simulink model "rfi_blk_v6_casper"  (Simulink 7.1)
settings: Solver=ode45  StartTime=0.0  StopTime=10.0  RelTol=1e-3
undefined symbols (not defined in the file): a, b
libraries: xbsIndex_r4 x13084, xps_library x4
(part 2 of 51)

== system rfi_blk_v6_casper/rfi_blk/mad_comp/sync_control ==
  note: 1K window acc_reset signal
  pps : Inport
  Constant : xbsIndex_r4/Constant  arith_type=Unsigned  const=1021  n_bits=10  bin_pt=0  explicit_period=on  period=1  equ=P=C  opselect=C  inp2=PCIN>>17  opr=+  inp1=P  carry=CIN  dbl_ovrd=off  block_type=constant
  Convert1 : xbsIndex_r4/Convert  arith_type=Boolean  n_bits=16  bin_pt=14  quantization=Truncate  overflow=Wrap  latency=0  dbl_ovrd=off  pipeline=off  block_type=convert
      settings: block_version=10.1.3
  Convert3 : xbsIndex_r4/Convert  arith_type=Boolean  n_bits=16  bin_pt=14  quantization=Truncate  overflow=Wrap  latency=0  dbl_ovrd=off  pipeline=off  block_type=convert
      settings: block_version=10.1.3
  Counter2 : xbsIndex_r4/Counter  cnt_type=Free Running  cnt_to=2048  operation=Up  start_count=0  cnt_by_val=1  arith_type=Unsigned  n_bits=10  bin_pt=0  load_pin=off  rst=off  en=off  explicit_period=on  period=1  dbl_ovrd=off  use_behavioral_HDL=off  implementation=Fabric  use_rpm=off  block_type=counter
  Delay2 : xbsIndex_r4/Delay  en=off  latency=1  dbl_ovrd=off  reg_retiming=off  block_type=delay
  Logical : xbsIndex_r4/Logical  logical_function=OR  inputs=2  en=off  latency=0  precision=Full  arith_type=Unsigned  n_bits=16  bin_pt=0  align_bp=on  dbl_ovrd=off  block_type=logical
  Relational : xbsIndex_r4/Relational  mode=a=b  en=off  latency=1  dbl_ovrd=off  block_type=relational
      settings: block_version=10.1.3
  Out1 : Outport
  wires:
  Counter2/1 -> Relational/1
  Constant/1 -> Relational/2
  Relational/1 -> Convert3/1
  Convert3/1 -> Logical/2
  Logical/1 -> Out1/1
  pps/1 -> Delay2/1
  Convert1/1 -> Logical/1
  Delay2/1 -> Convert1/1

== system rfi_blk_v6_casper/rfi_blk/cr1/cr/clip_repl ==
  note: 0: Bypass
      | 1: Replace by constant value
      | 2: Replace by median value
      | 3: Replace with Digital Noise
  p_thres : Inport  OutDataType=sfix(16)  OutScaling=2^0
  n_thres : Inport  Port=2  OutDataType=sfix(16)  OutScaling=2^0
  input : Inport  Port=3  OutDataType=sfix(16)  OutScaling=2^0
  median : Inport  Port=4  OutDataType=sfix(16)  OutScaling=2^0
  sigma_mad : Inport  Port=5  OutDataType=sfix(16)  OutScaling=2^0
  repl_val : Inport  Port=6  OutDataType=sfix(16)  OutScaling=2^0
  bypass_mux : Inport  Port=7  OutDataType=sfix(16)  OutScaling=2^0
  Delay1 : xbsIndex_r4/Delay  en=off  latency=3  dbl_ovrd=off  reg_retiming=off  block_type=delay
  Delay2 : xbsIndex_r4/Delay  en=off  latency=2  dbl_ovrd=off  reg_retiming=off  block_type=delay
  Delay3 : xbsIndex_r4/Delay  en=off  latency=2  dbl_ovrd=off  reg_retiming=off  block_type=delay
  Logical : xbsIndex_r4/Logical  logical_function=OR  inputs=2  en=off  latency=1  precision=Full  arith_type="Signed  (2's comp)"  n_bits=8  bin_pt=0  align_bp=on  dbl_ovrd=off  block_type=logical
  Mux : xbsIndex_r4/Mux  inputs=2  en=off  latency=1  precision=User Defined  arith_type="Signed  (2's comp)"  n_bits=8  bin_pt=0  quantization=Truncate  overflow=Wrap  dbl_ovrd=off  block_type=mux
  Mux1 : xbsIndex_r4/Mux  inputs=4  en=off  latency=1  precision=User Defined  arith_type="Signed  (2's comp)"  n_bits=8  bin_pt=0  quantization=Truncate  overflow=Wrap  dbl_ovrd=off  block_type=mux
  Mux2 : xbsIndex_r4/Mux  inputs=2  en=off  latency=1  precision=Full  arith_type="Signed  (2's comp)"  n_bits=8  bin_pt=0  quantization=Truncate  overflow=Wrap  dbl_ovrd=off  block_type=mux
  Mux3 : xbsIndex_r4/Mux  inputs=2  en=off  latency=1  precision=Full  arith_type="Signed  (2's comp)"  n_bits=8  bin_pt=0  quantization=Truncate  overflow=Wrap  dbl_ovrd=off  block_type=mux
  Relational : xbsIndex_r4/Relational  mode=a>b  en=off  latency=1  dbl_ovrd=off  block_type=relational
      settings: block_version=10.1.3
  Relational1 : xbsIndex_r4/Relational  mode=a<b  en=off  latency=1  dbl_ovrd=off  block_type=relational
      settings: block_version=10.1.3
  dns : Subsystem
  filter_o : Outport
  detect_o : Outport  Port=2
  input_data : Outport  Port=3
  wires:
  p_thres/1 -> Relational/2
  n_thres/1 -> Relational1/2
  Relational/1 -> Logical/1
  Relational1/1 -> Logical/2
  Logical/1 -> Mux/1, Mux2/1, Mux3/1, Delay2/1
  Delay1/1 -> Mux/2, Mux3/2, Mux1/2, Mux2/2, Delay3/1
  input/1 -> Relational/1, Relational1/1, Delay1/1
  Mux/1 -> Mux1/3
  Mux1/1 -> filter_o/1
  Mux3/1 -> Mux1/4
  Mux2/1 -> Mux1/5
  median/1 -> Mux3/3
  dns/1(dns_o) -> Mux2/3
  sigma_mad/1 -> dns/1(sigma_mad)
  Delay2/1 -> detect_o/1
  Delay3/1 -> input_data/1
  repl_val/1 -> Mux/3
  bypass_mux/1 -> Mux1/1

== system rfi_blk_v6_casper/rfi_blk/cr1/cr/thres_calc ==
  mad : Inport
  median : Inport  Port=2
  thres_fact : Inport  Port=3
  AddSub : xbsIndex_r4/AddSub  mode=Addition  use_carryin=off  use_carryout=off  en=off  latency=0  precision=Full  arith_type="Signed  (2's comp)"  n_bits=8  bin_pt=0  quantization=Truncate  overflow=Wrap  dbl_ovrd=off  use_behavioral_HDL=off  hw_selection=Fabric  pipelined=off  use_rpm=on  block_type=addsub
      settings: block_version=10.1.3
  AddSub1 : xbsIndex_r4/AddSub  mode=Subtraction  use_carryin=off  use_carryout=off  en=off  latency=0  precision=Full  arith_type="Signed  (2's comp)"  n_bits=8  bin_pt=0  quantization=Truncate  overflow=Wrap  dbl_ovrd=off  use_behavioral_HDL=off  hw_selection=Fabric  pipelined=off  use_rpm=on  block_type=addsub
      settings: block_version=10.1.3
  Constant : xbsIndex_r4/Constant  arith_type=Unsigned  const=1.4826  n_bits=8  bin_pt=7  explicit_period=on  period=1  equ=P=C  opselect=C  inp2=PCIN>>17  opr=+  inp1=P  carry=CIN  dbl_ovrd=off  block_type=constant
  Mult : xbsIndex_r4/Mult  precision=Full  arith_type="Signed  (2's comp)"  n_bits=16  bin_pt=14  quantization=Truncate  overflow=Wrap  en=off  latency=3  dbl_ovrd=off  use_behavioral_HDL=off  opt=Speed  use_embedded=on  optimum_pipeline=off  pipeline=on  use_rpm=on  placement_style=Triangular  block_type=mult
      settings: infoedit=Hardware notes: To use the internal pipeline stage of the dedicated multiplier you must select 'Pipeline for maximum performance'.  block_version=10.1.3
  Mult1 : xbsIndex_r4/Mult  precision=Full  arith_type=Unsigned  n_bits=8  bin_pt=0  quantization=Truncate  overflow=Wrap  en=off  latency=3  dbl_ovrd=off  use_behavioral_HDL=off  opt=Speed  use_embedded=on  optimum_pipeline=off  pipeline=on  use_rpm=on  placement_style=Triangular  block_type=mult
      settings: block_version=10.1.3
  Reinterpret : xbsIndex_r4/Reinterpret  force_arith_type=on  arith_type=Unsigned  force_bin_pt=off  bin_pt=0  block_type=reinterpret
      settings: infoedit="Changes signal type without altering the binary representation.   You can changed the signal between signed and unsigned, and relocate the binary point.<br><br>Hardware notes: In hardware this block costs nothing.<br><br>Example:  Suppose the input is 6 bits wide, signed, with 2 fractional bits, and the output is forced to unsigned with 0 fractional bits.  Then an input of -2.0 (1110.00 in binary 2's complement) becomes an output of 56 (111000 in binary)."  block_version=10.1.3
  Reinterpret1 : xbsIndex_r4/Reinterpret  force_arith_type=on  arith_type=Unsigned  force_bin_pt=off  bin_pt=0  block_type=reinterpret
      settings: infoedit="Changes signal type without altering the binary representation.   You can changed the signal between signed and unsigned, and relocate the binary point.<br><br>Hardware notes: In hardware this block costs nothing.<br><br>Example:  Suppose the input is 6 bits wide, signed, with 2 fractional bits, and the output is forced to unsigned with 0 fractional bits.  Then an input of -2.0 (1110.00 in binary 2's complement) becomes an output of 56 (111000 in binary)."  block_version=10.1.3
  Reinterpret2 : xbsIndex_r4/Reinterpret  force_arith_type=on  arith_type="Signed  (2's comp)"  force_bin_pt=off  bin_pt=0  block_type=reinterpret
      settings: infoedit="Changes signal type without altering the binary representation.   You can changed the signal between signed and unsigned, and relocate the binary point.<br><br>Hardware notes: In hardware this block costs nothing.<br><br>Example:  Suppose the input is 6 bits wide, signed, with 2 fractional bits, and the output is forced to unsigned with 0 fractional bits.  Then an input of -2.0 (1110.00 in binary 2's complement) becomes an output of 56 (111000 in binary)."  block_version=10.1.3
  Scope1 : Scope  NumInputPorts=5
      settings: YMin=-5~-5~-5~-5~-5  YMax=5~5~5~5~5  SaveName=ScopeData26
  Scope3 : Scope  NumInputPorts=5
      settings: YMin=-5~-5~-5~-5~-5  YMax=5~5~5~5~5  SaveName=ScopeData20
  p_th : Outport
  n_th : Outport  Port=2
  sigma_mad_o : Outport  Port=3
  wires:
  Constant/1 -> Mult/2
  Mult/1 -> Reinterpret/1
  Reinterpret/1 -> Mult1/1, sigma_mad_o/1
  Mult1/1 -> Reinterpret1/1
  mad/1 -> Mult/1, Scope3/2
  median/1 -> Reinterpret2/1, Scope3/1
  Reinterpret1/1 -> AddSub/2, AddSub1/2
  AddSub/1 -> p_th/1, Scope1/1
  AddSub1/1 -> n_th/1, Scope1/2
  Reinterpret2/1 -> AddSub/1, AddSub1/1
  thres_fact/1 -> Mult1/2

== system rfi_blk_v6_casper/rfi_blk/cr2/cr/clip_repl ==
  note: 0: Bypass
      | 1: Replace by constant value
      | 2: Replace by median value
      | 3: Replace with Digital Noise
  p_thres : Inport  OutDataType=sfix(16)  OutScaling=2^0
  n_thres : Inport  Port=2  OutDataType=sfix(16)  OutScaling=2^0
  input : Inport  Port=3  OutDataType=sfix(16)  OutScaling=2^0
  median : Inport  Port=4  OutDataType=sfix(16)  OutScaling=2^0
  sigma_mad : Inport  Port=5  OutDataType=sfix(16)  OutScaling=2^0
  repl_val : Inport  Port=6  OutDataType=sfix(16)  OutScaling=2^0
  bypass_mux : Inport  Port=7  OutDataType=sfix(16)  OutScaling=2^0
  Delay1 : xbsIndex_r4/Delay  en=off  latency=3  dbl_ovrd=off  reg_retiming=off  block_type=delay
  Delay2 : xbsIndex_r4/Delay  en=off  latency=2  dbl_ovrd=off  reg_retiming=off  block_type=delay
  Delay3 : xbsIndex_r4/Delay  en=off  latency=2  dbl_ovrd=off  reg_retiming=off  block_type=delay
  Logical : xbsIndex_r4/Logical  logical_function=OR  inputs=2  en=off  latency=1  precision=Full  arith_type="Signed  (2's comp)"  n_bits=8  bin_pt=0  align_bp=on  dbl_ovrd=off  block_type=logical
  Mux : xbsIndex_r4/Mux  inputs=2  en=off  latency=1  precision=User Defined  arith_type="Signed  (2's comp)"  n_bits=8  bin_pt=0  quantization=Truncate  overflow=Wrap  dbl_ovrd=off  block_type=mux
  Mux1 : xbsIndex_r4/Mux  inputs=4  en=off  latency=1  precision=User Defined  arith_type="Signed  (2's comp)"  n_bits=8  bin_pt=0  quantization=Truncate  overflow=Wrap  dbl_ovrd=off  block_type=mux
  Mux2 : xbsIndex_r4/Mux  inputs=2  en=off  latency=1  precision=Full  arith_type="Signed  (2's comp)"  n_bits=8  bin_pt=0  quantization=Truncate  overflow=Wrap  dbl_ovrd=off  block_type=mux
  Mux3 : xbsIndex_r4/Mux  inputs=2  en=off  latency=1  precision=Full  arith_type="Signed  (2's comp)"  n_bits=8  bin_pt=0  quantization=Truncate  overflow=Wrap  dbl_ovrd=off  block_type=mux
  Relational : xbsIndex_r4/Relational  mode=a>b  en=off  latency=1  dbl_ovrd=off  block_type=relational
      settings: block_version=10.1.3
  Relational1 : xbsIndex_r4/Relational  mode=a<b  en=off  latency=1  dbl_ovrd=off  block_type=relational
      settings: block_version=10.1.3
  dns : Subsystem
  filter_o : Outport
  detect_o : Outport  Port=2
  input_data : Outport  Port=3
  wires:
  p_thres/1 -> Relational/2
  n_thres/1 -> Relational1/2
  Relational/1 -> Logical/1
  Relational1/1 -> Logical/2
  Logical/1 -> Mux/1, Mux2/1, Mux3/1, Delay2/1
  Delay1/1 -> Mux/2, Mux3/2, Mux1/2, Mux2/2, Delay3/1
  input/1 -> Relational/1, Relational1/1, Delay1/1
  Mux/1 -> Mux1/3
  Mux1/1 -> filter_o/1
  Mux3/1 -> Mux1/4
  Mux2/1 -> Mux1/5
  median/1 -> Mux3/3
  dns/1(dns_o) -> Mux2/3
  sigma_mad/1 -> dns/1(sigma_mad)
  Delay2/1 -> detect_o/1
  Delay3/1 -> input_data/1
  repl_val/1 -> Mux/3
  bypass_mux/1 -> Mux1/1

== system rfi_blk_v6_casper/rfi_blk/cr2/cr/thres_calc ==
  mad : Inport
  median : Inport  Port=2
  thres_fact : Inport  Port=3
  AddSub : xbsIndex_r4/AddSub  mode=Addition  use_carryin=off  use_carryout=off  en=off  latency=0  precision=Full  arith_type="Signed  (2's comp)"  n_bits=8  bin_pt=0  quantization=Truncate  overflow=Wrap  dbl_ovrd=off  use_behavioral_HDL=off  hw_selection=Fabric  pipelined=off  use_rpm=on  block_type=addsub
      settings: block_version=10.1.3
  AddSub1 : xbsIndex_r4/AddSub  mode=Subtraction  use_carryin=off  use_carryout=off  en=off  latency=0  precision=Full  arith_type="Signed  (2's comp)"  n_bits=8  bin_pt=0  quantization=Truncate  overflow=Wrap  dbl_ovrd=off  use_behavioral_HDL=off  hw_selection=Fabric  pipelined=off  use_rpm=on  block_type=addsub
      settings: block_version=10.1.3
  Constant : xbsIndex_r4/Constant  arith_type=Unsigned  const=1.4826  n_bits=8  bin_pt=7  explicit_period=on  period=1  equ=P=C  opselect=C  inp2=PCIN>>17  opr=+  inp1=P  carry=CIN  dbl_ovrd=off  block_type=constant
  Mult : xbsIndex_r4/Mult  precision=Full  arith_type="Signed  (2's comp)"  n_bits=16  bin_pt=14  quantization=Truncate  overflow=Wrap  en=off  latency=3  dbl_ovrd=off  use_behavioral_HDL=off  opt=Speed  use_embedded=on  optimum_pipeline=off  pipeline=on  use_rpm=on  placement_style=Triangular  block_type=mult
      settings: infoedit=Hardware notes: To use the internal pipeline stage of the dedicated multiplier you must select 'Pipeline for maximum performance'.  block_version=10.1.3
  Mult1 : xbsIndex_r4/Mult  precision=Full  arith_type=Unsigned  n_bits=8  bin_pt=0  quantization=Truncate  overflow=Wrap  en=off  latency=3  dbl_ovrd=off  use_behavioral_HDL=off  opt=Speed  use_embedded=on  optimum_pipeline=off  pipeline=on  use_rpm=on  placement_style=Triangular  block_type=mult
      settings: block_version=10.1.3
  Reinterpret : xbsIndex_r4/Reinterpret  force_arith_type=on  arith_type=Unsigned  force_bin_pt=off  bin_pt=0  block_type=reinterpret
      settings: infoedit="Changes signal type without altering the binary representation.   You can changed the signal between signed and unsigned, and relocate the binary point.<br><br>Hardware notes: In hardware this block costs nothing.<br><br>Example:  Suppose the input is 6 bits wide, signed, with 2 fractional bits, and the output is forced to unsigned with 0 fractional bits.  Then an input of -2.0 (1110.00 in binary 2's complement) becomes an output of 56 (111000 in binary)."  block_version=10.1.3
  Reinterpret1 : xbsIndex_r4/Reinterpret  force_arith_type=on  arith_type=Unsigned  force_bin_pt=off  bin_pt=0  block_type=reinterpret
      settings: infoedit="Changes signal type without altering the binary representation.   You can changed the signal between signed and unsigned, and relocate the binary point.<br><br>Hardware notes: In hardware this block costs nothing.<br><br>Example:  Suppose the input is 6 bits wide, signed, with 2 fractional bits, and the output is forced to unsigned with 0 fractional bits.  Then an input of -2.0 (1110.00 in binary 2's complement) becomes an output of 56 (111000 in binary)."  block_version=10.1.3
  Reinterpret2 : xbsIndex_r4/Reinterpret  force_arith_type=on  arith_type="Signed  (2's comp)"  force_bin_pt=off  bin_pt=0  block_type=reinterpret
      settings: infoedit="Changes signal type without altering the binary representation.   You can changed the signal between signed and unsigned, and relocate the binary point.<br><br>Hardware notes: In hardware this block costs nothing.<br><br>Example:  Suppose the input is 6 bits wide, signed, with 2 fractional bits, and the output is forced to unsigned with 0 fractional bits.  Then an input of -2.0 (1110.00 in binary 2's complement) becomes an output of 56 (111000 in binary)."  block_version=10.1.3
  Scope1 : Scope  NumInputPorts=5
      settings: YMin=-5~-5~-5~-5~-5  YMax=5~5~5~5~5  SaveName=ScopeData26
  Scope3 : Scope  NumInputPorts=5
      settings: YMin=-5~-5~-5~-5~-5  YMax=5~5~5~5~5  SaveName=ScopeData20
  p_th : Outport
  n_th : Outport  Port=2
  sigma_mad_o : Outport  Port=3
  wires:
  Constant/1 -> Mult/2
  Mult/1 -> Reinterpret/1
  Reinterpret/1 -> Mult1/1, sigma_mad_o/1
  Mult1/1 -> Reinterpret1/1
  mad/1 -> Mult/1, Scope3/2
  median/1 -> Reinterpret2/1, Scope3/1
  Reinterpret1/1 -> AddSub/2, AddSub1/2
  AddSub/1 -> p_th/1, Scope1/1
  AddSub1/1 -> n_th/1, Scope1/2
  Reinterpret2/1 -> AddSub/1, AddSub1/1
  thres_fact/1 -> Mult1/2

== system rfi_blk_v6_casper/rfi_blk/cr4/cr/clip_repl ==
  note: 0: Bypass
      | 1: Replace by constant value
      | 2: Replace by median value
      | 3: Replace with Digital Noise
  p_thres : Inport  OutDataType=sfix(16)  OutScaling=2^0
  n_thres : Inport  Port=2  OutDataType=sfix(16)  OutScaling=2^0
  input : Inport  Port=3  OutDataType=sfix(16)  OutScaling=2^0
  median : Inport  Port=4  OutDataType=sfix(16)  OutScaling=2^0
  sigma_mad : Inport  Port=5  OutDataType=sfix(16)  OutScaling=2^0
  repl_val : Inport  Port=6  OutDataType=sfix(16)  OutScaling=2^0
  bypass_mux : Inport  Port=7  OutDataType=sfix(16)  OutScaling=2^0
  Delay1 : xbsIndex_r4/Delay  en=off  latency=3  dbl_ovrd=off  reg_retiming=off  block_type=delay
  Delay2 : xbsIndex_r4/Delay  en=off  latency=2  dbl_ovrd=off  reg_retiming=off  block_type=delay
  Delay3 : xbsIndex_r4/Delay  en=off  latency=2  dbl_ovrd=off  reg_retiming=off  block_type=delay
  Logical : xbsIndex_r4/Logical  logical_function=OR  inputs=2  en=off  latency=1  precision=Full  arith_type="Signed  (2's comp)"  n_bits=8  bin_pt=0  align_bp=on  dbl_ovrd=off  block_type=logical
  Mux : xbsIndex_r4/Mux  inputs=2  en=off  latency=1  precision=User Defined  arith_type="Signed  (2's comp)"  n_bits=8  bin_pt=0  quantization=Truncate  overflow=Wrap  dbl_ovrd=off  block_type=mux
  Mux1 : xbsIndex_r4/Mux  inputs=4  en=off  latency=1  precision=User Defined  arith_type="Signed  (2's comp)"  n_bits=8  bin_pt=0  quantization=Truncate  overflow=Wrap  dbl_ovrd=off  block_type=mux
  Mux2 : xbsIndex_r4/Mux  inputs=2  en=off  latency=1  precision=Full  arith_type="Signed  (2's comp)"  n_bits=8  bin_pt=0  quantization=Truncate  overflow=Wrap  dbl_ovrd=off  block_type=mux
  Mux3 : xbsIndex_r4/Mux  inputs=2  en=off  latency=1  precision=Full  arith_type="Signed  (2's comp)"  n_bits=8  bin_pt=0  quantization=Truncate  overflow=Wrap  dbl_ovrd=off  block_type=mux
  Relational : xbsIndex_r4/Relational  mode=a>b  en=off  latency=1  dbl_ovrd=off  block_type=relational
      settings: block_version=10.1.3
  Relational1 : xbsIndex_r4/Relational  mode=a<b  en=off  latency=1  dbl_ovrd=off  block_type=relational
      settings: block_version=10.1.3
  dns : Subsystem
  filter_o : Outport
  detect_o : Outport  Port=2
  input_data : Outport  Port=3
  wires:
  p_thres/1 -> Relational/2
  n_thres/1 -> Relational1/2
  Relational/1 -> Logical/1
  Relational1/1 -> Logical/2
  Logical/1 -> Mux/1, Mux2/1, Mux3/1, Delay2/1
  Delay1/1 -> Mux/2, Mux3/2, Mux1/2, Mux2/2, Delay3/1
  input/1 -> Relational/1, Relational1/1, Delay1/1
  Mux/1 -> Mux1/3
  Mux1/1 -> filter_o/1
  Mux3/1 -> Mux1/4
  Mux2/1 -> Mux1/5
  median/1 -> Mux3/3
  dns/1(dns_o) -> Mux2/3
  sigma_mad/1 -> dns/1(sigma_mad)
  Delay2/1 -> detect_o/1
  Delay3/1 -> input_data/1
  repl_val/1 -> Mux/3
  bypass_mux/1 -> Mux1/1

== system rfi_blk_v6_casper/rfi_blk/cr4/cr/thres_calc ==
  mad : Inport
  median : Inport  Port=2
  thres_fact : Inport  Port=3
  AddSub : xbsIndex_r4/AddSub  mode=Addition  use_carryin=off  use_carryout=off  en=off  latency=0  precision=Full  arith_type="Signed  (2's comp)"  n_bits=8  bin_pt=0  quantization=Truncate  overflow=Wrap  dbl_ovrd=off  use_behavioral_HDL=off  hw_selection=Fabric  pipelined=off  use_rpm=on  block_type=addsub
      settings: block_version=10.1.3
  AddSub1 : xbsIndex_r4/AddSub  mode=Subtraction  use_carryin=off  use_carryout=off  en=off  latency=0  precision=Full  arith_type="Signed  (2's comp)"  n_bits=8  bin_pt=0  quantization=Truncate  overflow=Wrap  dbl_ovrd=off  use_behavioral_HDL=off  hw_selection=Fabric  pipelined=off  use_rpm=on  block_type=addsub
      settings: block_version=10.1.3
  Constant : xbsIndex_r4/Constant  arith_type=Unsigned  const=1.4826  n_bits=8  bin_pt=7  explicit_period=on  period=1  equ=P=C  opselect=C  inp2=PCIN>>17  opr=+  inp1=P  carry=CIN  dbl_ovrd=off  block_type=constant
  Mult : xbsIndex_r4/Mult  precision=Full  arith_type="Signed  (2's comp)"  n_bits=16  bin_pt=14  quantization=Truncate  overflow=Wrap  en=off  latency=3  dbl_ovrd=off  use_behavioral_HDL=off  opt=Speed  use_embedded=on  optimum_pipeline=off  pipeline=on  use_rpm=on  placement_style=Triangular  block_type=mult
      settings: infoedit=Hardware notes: To use the internal pipeline stage of the dedicated multiplier you must select 'Pipeline for maximum performance'.  block_version=10.1.3
  Mult1 : xbsIndex_r4/Mult  precision=Full  arith_type=Unsigned  n_bits=8  bin_pt=0  quantization=Truncate  overflow=Wrap  en=off  latency=3  dbl_ovrd=off  use_behavioral_HDL=off  opt=Speed  use_embedded=on  optimum_pipeline=off  pipeline=on  use_rpm=on  placement_style=Triangular  block_type=mult
      settings: block_version=10.1.3
  Reinterpret : xbsIndex_r4/Reinterpret  force_arith_type=on  arith_type=Unsigned  force_bin_pt=off  bin_pt=0  block_type=reinterpret
      settings: infoedit="Changes signal type without altering the binary representation.   You can changed the signal between signed and unsigned, and relocate the binary point.<br><br>Hardware notes: In hardware this block costs nothing.<br><br>Example:  Suppose the input is 6 bits wide, signed, with 2 fractional bits, and the output is forced to unsigned with 0 fractional bits.  Then an input of -2.0 (1110.00 in binary 2's complement) becomes an output of 56 (111000 in binary)."  block_version=10.1.3
  Reinterpret1 : xbsIndex_r4/Reinterpret  force_arith_type=on  arith_type=Unsigned  force_bin_pt=off  bin_pt=0  block_type=reinterpret
      settings: infoedit="Changes signal type without altering the binary representation.   You can changed the signal between signed and unsigned, and relocate the binary point.<br><br>Hardware notes: In hardware this block costs nothing.<br><br>Example:  Suppose the input is 6 bits wide, signed, with 2 fractional bits, and the output is forced to unsigned with 0 fractional bits.  Then an input of -2.0 (1110.00 in binary 2's complement) becomes an output of 56 (111000 in binary)."  block_version=10.1.3
  Reinterpret2 : xbsIndex_r4/Reinterpret  force_arith_type=on  arith_type="Signed  (2's comp)"  force_bin_pt=off  bin_pt=0  block_type=reinterpret
      settings: infoedit="Changes signal type without altering the binary representation.   You can changed the signal between signed and unsigned, and relocate the binary point.<br><br>Hardware notes: In hardware this block costs nothing.<br><br>Example:  Suppose the input is 6 bits wide, signed, with 2 fractional bits, and the output is forced to unsigned with 0 fractional bits.  Then an input of -2.0 (1110.00 in binary 2's complement) becomes an output of 56 (111000 in binary)."  block_version=10.1.3
  Scope1 : Scope  NumInputPorts=5
      settings: YMin=-5~-5~-5~-5~-5  YMax=5~5~5~5~5  SaveName=ScopeData26
  Scope3 : Scope  NumInputPorts=5
      settings: YMin=-5~-5~-5~-5~-5  YMax=5~5~5~5~5  SaveName=ScopeData20
  p_th : Outport
  n_th : Outport  Port=2
  sigma_mad_o : Outport  Port=3
  wires:
  Constant/1 -> Mult/2
  Mult/1 -> Reinterpret/1
  Reinterpret/1 -> Mult1/1, sigma_mad_o/1
  Mult1/1 -> Reinterpret1/1
  mad/1 -> Mult/1, Scope3/2
  median/1 -> Reinterpret2/1, Scope3/1
  Reinterpret1/1 -> AddSub/2, AddSub1/2
  AddSub/1 -> p_th/1, Scope1/1
  AddSub1/1 -> n_th/1, Scope1/2
  Reinterpret2/1 -> AddSub/1, AddSub1/1
  thres_fact/1 -> Mult1/2

== system rfi_blk_v6_casper/rfi_blk/cr5/cr/clip_repl ==
  note: 0: Bypass
      | 1: Replace by constant value
      | 2: Replace by median value
      | 3: Replace with Digital Noise
  p_thres : Inport  OutDataType=sfix(16)  OutScaling=2^0
  n_thres : Inport  Port=2  OutDataType=sfix(16)  OutScaling=2^0
  input : Inport  Port=3  OutDataType=sfix(16)  OutScaling=2^0
  median : Inport  Port=4  OutDataType=sfix(16)  OutScaling=2^0
  sigma_mad : Inport  Port=5  OutDataType=sfix(16)  OutScaling=2^0
  repl_val : Inport  Port=6  OutDataType=sfix(16)  OutScaling=2^0
  bypass_mux : Inport  Port=7  OutDataType=sfix(16)  OutScaling=2^0
  Delay1 : xbsIndex_r4/Delay  en=off  latency=3  dbl_ovrd=off  reg_retiming=off  block_type=delay
  Delay2 : xbsIndex_r4/Delay  en=off  latency=2  dbl_ovrd=off  reg_retiming=off  block_type=delay
  Delay3 : xbsIndex_r4/Delay  en=off  latency=2  dbl_ovrd=off  reg_retiming=off  block_type=delay
  Logical : xbsIndex_r4/Logical  logical_function=OR  inputs=2  en=off  latency=1  precision=Full  arith_type="Signed  (2's comp)"  n_bits=8  bin_pt=0  align_bp=on  dbl_ovrd=off  block_type=logical
  Mux : xbsIndex_r4/Mux  inputs=2  en=off  latency=1  precision=User Defined  arith_type="Signed  (2's comp)"  n_bits=8  bin_pt=0  quantization=Truncate  overflow=Wrap  dbl_ovrd=off  block_type=mux
  Mux1 : xbsIndex_r4/Mux  inputs=4  en=off  latency=1  precision=User Defined  arith_type="Signed  (2's comp)"  n_bits=8  bin_pt=0  quantization=Truncate  overflow=Wrap  dbl_ovrd=off  block_type=mux
  Mux2 : xbsIndex_r4/Mux  inputs=2  en=off  latency=1  precision=Full  arith_type="Signed  (2's comp)"  n_bits=8  bin_pt=0  quantization=Truncate  overflow=Wrap  dbl_ovrd=off  block_type=mux
  Mux3 : xbsIndex_r4/Mux  inputs=2  en=off  latency=1  precision=Full  arith_type="Signed  (2's comp)"  n_bits=8  bin_pt=0  quantization=Truncate  overflow=Wrap  dbl_ovrd=off  block_type=mux
  Relational : xbsIndex_r4/Relational  mode=a>b  en=off  latency=1  dbl_ovrd=off  block_type=relational
      settings: block_version=10.1.3
  Relational1 : xbsIndex_r4/Relational  mode=a<b  en=off  latency=1  dbl_ovrd=off  block_type=relational
      settings: block_version=10.1.3
  dns : Subsystem
  filter_o : Outport
  detect_o : Outport  Port=2
  input_data : Outport  Port=3
  wires:
  p_thres/1 -> Relational/2
  n_thres/1 -> Relational1/2
  Relational/1 -> Logical/1
  Relational1/1 -> Logical/2
  Logical/1 -> Mux/1, Mux2/1, Mux3/1, Delay2/1
  Delay1/1 -> Mux/2, Mux3/2, Mux1/2, Mux2/2, Delay3/1
  input/1 -> Relational/1, Relational1/1, Delay1/1
  Mux/1 -> Mux1/3
  Mux1/1 -> filter_o/1
  Mux3/1 -> Mux1/4
  Mux2/1 -> Mux1/5
  median/1 -> Mux3/3
  dns/1(dns_o) -> Mux2/3
  sigma_mad/1 -> dns/1(sigma_mad)
  Delay2/1 -> detect_o/1
  Delay3/1 -> input_data/1
  repl_val/1 -> Mux/3
  bypass_mux/1 -> Mux1/1

== system rfi_blk_v6_casper/rfi_blk/cr5/cr/thres_calc ==
  mad : Inport
  median : Inport  Port=2
  thres_fact : Inport  Port=3
  AddSub : xbsIndex_r4/AddSub  mode=Addition  use_carryin=off  use_carryout=off  en=off  latency=0  precision=Full  arith_type="Signed  (2's comp)"  n_bits=8  bin_pt=0  quantization=Truncate  overflow=Wrap  dbl_ovrd=off  use_behavioral_HDL=off  hw_selection=Fabric  pipelined=off  use_rpm=on  block_type=addsub
      settings: block_version=10.1.3
  AddSub1 : xbsIndex_r4/AddSub  mode=Subtraction  use_carryin=off  use_carryout=off  en=off  latency=0  precision=Full  arith_type="Signed  (2's comp)"  n_bits=8  bin_pt=0  quantization=Truncate  overflow=Wrap  dbl_ovrd=off  use_behavioral_HDL=off  hw_selection=Fabric  pipelined=off  use_rpm=on  block_type=addsub
      settings: block_version=10.1.3
  Constant : xbsIndex_r4/Constant  arith_type=Unsigned  const=1.4826  n_bits=8  bin_pt=7  explicit_period=on  period=1  equ=P=C  opselect=C  inp2=PCIN>>17  opr=+  inp1=P  carry=CIN  dbl_ovrd=off  block_type=constant
  Mult : xbsIndex_r4/Mult  precision=Full  arith_type="Signed  (2's comp)"  n_bits=16  bin_pt=14  quantization=Truncate  overflow=Wrap  en=off  latency=3  dbl_ovrd=off  use_behavioral_HDL=off  opt=Speed  use_embedded=on  optimum_pipeline=off  pipeline=on  use_rpm=on  placement_style=Triangular  block_type=mult
      settings: infoedit=Hardware notes: To use the internal pipeline stage of the dedicated multiplier you must select 'Pipeline for maximum performance'.  block_version=10.1.3
  Mult1 : xbsIndex_r4/Mult  precision=Full  arith_type=Unsigned  n_bits=8  bin_pt=0  quantization=Truncate  overflow=Wrap  en=off  latency=3  dbl_ovrd=off  use_behavioral_HDL=off  opt=Speed  use_embedded=on  optimum_pipeline=off  pipeline=on  use_rpm=on  placement_style=Triangular  block_type=mult
      settings: block_version=10.1.3
  Reinterpret : xbsIndex_r4/Reinterpret  force_arith_type=on  arith_type=Unsigned  force_bin_pt=off  bin_pt=0  block_type=reinterpret
      settings: infoedit="Changes signal type without altering the binary representation.   You can changed the signal between signed and unsigned, and relocate the binary point.<br><br>Hardware notes: In hardware this block costs nothing.<br><br>Example:  Suppose the input is 6 bits wide, signed, with 2 fractional bits, and the output is forced to unsigned with 0 fractional bits.  Then an input of -2.0 (1110.00 in binary 2's complement) becomes an output of 56 (111000 in binary)."  block_version=10.1.3
  Reinterpret1 : xbsIndex_r4/Reinterpret  force_arith_type=on  arith_type=Unsigned  force_bin_pt=off  bin_pt=0  block_type=reinterpret
      settings: infoedit="Changes signal type without altering the binary representation.   You can changed the signal between signed and unsigned, and relocate the binary point.<br><br>Hardware notes: In hardware this block costs nothing.<br><br>Example:  Suppose the input is 6 bits wide, signed, with 2 fractional bits, and the output is forced to unsigned with 0 fractional bits.  Then an input of -2.0 (1110.00 in binary 2's complement) becomes an output of 56 (111000 in binary)."  block_version=10.1.3
  Reinterpret2 : xbsIndex_r4/Reinterpret  force_arith_type=on  arith_type="Signed  (2's comp)"  force_bin_pt=off  bin_pt=0  block_type=reinterpret
      settings: infoedit="Changes signal type without altering the binary representation.   You can changed the signal between signed and unsigned, and relocate the binary point.<br><br>Hardware notes: In hardware this block costs nothing.<br><br>Example:  Suppose the input is 6 bits wide, signed, with 2 fractional bits, and the output is forced to unsigned with 0 fractional bits.  Then an input of -2.0 (1110.00 in binary 2's complement) becomes an output of 56 (111000 in binary)."  block_version=10.1.3
  Scope1 : Scope  NumInputPorts=5
      settings: YMin=-5~-5~-5~-5~-5  YMax=5~5~5~5~5  SaveName=ScopeData26
  Scope3 : Scope  NumInputPorts=5
      settings: YMin=-5~-5~-5~-5~-5  YMax=5~5~5~5~5  SaveName=ScopeData20
  p_th : Outport
  n_th : Outport  Port=2
  sigma_mad_o : Outport  Port=3
  wires:
  Constant/1 -> Mult/2
  Mult/1 -> Reinterpret/1
  Reinterpret/1 -> Mult1/1, sigma_mad_o/1
  Mult1/1 -> Reinterpret1/1
  mad/1 -> Mult/1, Scope3/2
  median/1 -> Reinterpret2/1, Scope3/1
  Reinterpret1/1 -> AddSub/2, AddSub1/2
  AddSub/1 -> p_th/1, Scope1/1
  AddSub1/1 -> n_th/1, Scope1/2
  Reinterpret2/1 -> AddSub/1, AddSub1/1
  thres_fact/1 -> Mult1/2

== system rfi_blk_v6_casper/rfi_blk/mad_comp/Median1/Subsystem ==
  data_in : Inport  OutDataType=sfix(16)  OutScaling=2^0
  med_loc : Inport  Port=2  OutDataType=sfix(16)  OutScaling=2^0
  acc_reset : Inport  Port=3  OutDataType=sfix(16)  OutScaling=2^0
  " System Generator" : xbsIndex_r4/ System Generator  Tag=genX  SystemSampleTime=-1  xilinxfamily=Virtex4  part=xc4vsx35  speed=-10  package=ff668  synthesis_tool=XST  clock_wrapper=Clock Enables  directory=./netlist  testbench=off  simulink_period=1  sysclk_period=100  dcm_input_clock_period=100  incr_netlist=off  trim_vbits=Everywhere in SubSystem  dbl_ovrd=According to Block Masks  core_generation=According to Block Masks  run_coregen=off  deprecated_control=off  eval_field=0  block_type=sysgen
      settings: block_version=10.1.3
  Constant10 : xbsIndex_r4/Constant  arith_type=Boolean  const=1  n_bits=1  bin_pt=0  explicit_period=on  period=1  equ=P=C  opselect=C  inp2=PCIN>>17  opr=+  inp1=P  carry=CIN  dbl_ovrd=off  block_type=constant
  Constant11 : xbsIndex_r4/Constant  arith_type=Boolean  const=0  n_bits=1  bin_pt=0  explicit_period=on  period=1  equ=P=C  opselect=C  inp2=PCIN>>17  opr=+  inp1=P  carry=CIN  dbl_ovrd=off  block_type=constant
  Constant12 : xbsIndex_r4/Constant  arith_type=Boolean  const=1  n_bits=1  bin_pt=0  explicit_period=on  period=1  equ=P=C  opselect=C  inp2=PCIN>>17  opr=+  inp1=P  carry=CIN  dbl_ovrd=off  block_type=constant
  Constant2 : xbsIndex_r4/Constant  arith_type=Unsigned  const=0  n_bits=8  bin_pt=0  explicit_period=on  period=1  equ=P=C  opselect=C  inp2=PCIN>>17  opr=+  inp1=P  carry=CIN  dbl_ovrd=off  block_type=constant
  Constant3 : xbsIndex_r4/Constant  arith_type=Unsigned  const=0  n_bits=8  bin_pt=0  explicit_period=on  period=1  equ=P=C  opselect=C  inp2=PCIN>>17  opr=+  inp1=P  carry=CIN  dbl_ovrd=off  block_type=constant
  Constant9 : xbsIndex_r4/Constant  arith_type=Boolean  const=0  n_bits=1  bin_pt=0  explicit_period=on  period=1  equ=P=C  opselect=C  inp2=PCIN>>17  opr=+  inp1=P  carry=CIN  dbl_ovrd=off  block_type=constant
  Inverter : xbsIndex_r4/Inverter  en=off  latency=0  dbl_ovrd=off  block_type=inv
  Logical : xbsIndex_r4/Logical  logical_function=AND  inputs=2  en=off  latency=0  precision=Full  arith_type=Unsigned  n_bits=16  bin_pt=0  align_bp=on  dbl_ovrd=off  block_type=logical
  Logical1 : xbsIndex_r4/Logical  logical_function=OR  inputs=2  en=off  latency=1  precision=User Defined  arith_type=Unsigned  n_bits=1  bin_pt=0  align_bp=on  dbl_ovrd=off  block_type=logical
  Logical2 : xbsIndex_r4/Logical  logical_function=OR  inputs=2  en=off  latency=1  precision=User Defined  arith_type="Signed  (2's comp)"  n_bits=8  bin_pt=0  align_bp=on  dbl_ovrd=off  block_type=logical
  Median_Accumulator1 : Subsystem
  Median_Accumulator2 : Subsystem
  Median_Location1 : Subsystem
  Median_Location2 : Subsystem
  Mux : xbsIndex_r4/Mux  inputs=2  en=off  latency=0  precision=Full  arith_type=Unsigned  n_bits=16  bin_pt=14  quantization=Truncate  overflow=Wrap  dbl_ovrd=off  block_type=mux
  Mux1 : xbsIndex_r4/Mux  inputs=2  en=off  latency=0  precision=Full  arith_type="Signed  (2's comp)"  n_bits=8  bin_pt=0  quantization=Truncate  overflow=Wrap  dbl_ovrd=off  block_type=mux
  Mux2 : xbsIndex_r4/Mux  inputs=2  en=off  latency=0  precision=User Defined  arith_type="Signed  (2's comp)"  n_bits=8  bin_pt=0  quantization=Truncate  overflow=Wrap  dbl_ovrd=off  block_type=mux
  Mux3 : xbsIndex_r4/Mux  inputs=2  en=off  latency=0  precision=User Defined  arith_type="Signed  (2's comp)"  n_bits=8  bin_pt=0  quantization=Truncate  overflow=Wrap  dbl_ovrd=off  block_type=mux
  Scope{1..5} (5 alike) : Scope  NumInputPorts=2
      Scope1 settings: YMin=-5~-5  YMax=5~5
      Scope2 settings: YMin=-5~-5  YMax=5~5
      Scope3 settings: YMin=-5~-5  YMax=5~5  SaveName=ScopeData5
      Scope4 settings: YMin=-5~-5  YMax=5~5  SaveName=ScopeData7
      Scope5 settings: YMin=-5~-5  YMax=5~5  SaveName=ScopeData15
  priority_out1 : Outport  OutDataType=sfix(16)  OutScaling=2^0
  med_calc1 : Outport  Port=2  OutDataType=sfix(16)  OutScaling=2^0
  inc_out : Outport  Port=3
  wires:
  data_in/1 -> Median_Accumulator1/1(data_in), Median_Accumulator2/1(data_in)
  Median_Accumulator1/1(Out1) -> Median_Location1/1(In1), Scope5/1
  Median_Accumulator1/2(Out2) -> Median_Location1/2(In2)
  Median_Accumulator1/3(Out3) -> Median_Location1/3(In3)
  Median_Accumulator1/4(Out4) -> Median_Location1/4(In4)
  Median_Accumulator1/5(Out5) -> Median_Location1/5(In5), Scope5/2
  Median_Accumulator1/6(Out6) -> Median_Location1/6(In6)
  Median_Accumulator1/7(Out7) -> Median_Location1/7(In7)
  Median_Accumulator1/8(Out8) -> Median_Location1/8(In8)
  Median_Location1/1(Out1) -> Scope1/1, Mux2/3
  med_loc/1 -> Median_Location1/9(median_loc), Median_Location2/9(median_loc)
  Median_Accumulator2/8(Out8) -> Median_Location2/8(In8)
  Median_Accumulator2/7(Out7) -> Median_Location2/7(In7)
  Median_Accumulator2/6(Out6) -> Median_Location2/6(In6)
  Median_Accumulator2/5(Out5) -> Median_Location2/5(In5)
  Median_Accumulator2/4(Out4) -> Median_Location2/4(In4)
  Median_Accumulator2/3(Out3) -> Median_Location2/3(In3)
  Median_Accumulator2/2(Out2) -> Median_Location2/2(In2)
  Median_Accumulator2/1(Out1) -> Median_Location2/1(In1)
  Constant9/1 -> Mux/2
  Constant10/1 -> Mux/3
  Mux/1 -> Logical1/1, Mux2/1
  Inverter/1 -> Logical/1
  Logical/1 -> Mux1/1
  Constant11/1 -> Mux1/2
  Mux1/1 -> Logical1/2, Mux3/1
  Median_Location2/1(Out3) -> Mux3/3, Scope2/1, Scope4/2
  Constant12/1 -> Mux1/3
  Mux2/1 -> Logical2/1
  Mux3/1 -> Logical2/2
  Constant2/1 -> Mux2/2
  Constant3/1 -> Mux3/2
  Logical2/1 -> Scope3/2, med_calc1/1
  Logical1/1 -> priority_out1/1, Scope3/1
  Median_Accumulator2/9(inc_signal) -> inc_out/1
  Median_Location1/2(sel1) -> Mux/1, Inverter/1, Scope1/2
  Median_Location2/2(sel2) -> Logical/2, Scope2/2
  acc_reset/1 -> Median_Accumulator1/2(acc_reset), Median_Accumulator2/2(acc_reset)

== system rfi_blk_v6_casper/rfi_blk/mad_comp/Median1/Subsystem1 ==
  data_in : Inport  OutDataType=sfix(16)  OutScaling=2^0
  med_loc : Inport  Port=2  OutDataType=sfix(16)  OutScaling=2^0
  acc_reset : Inport  Port=3  OutDataType=sfix(16)  OutScaling=2^0
  " System Generator" : xbsIndex_r4/ System Generator  Tag=genX  SystemSampleTime=-1  xilinxfamily=Virtex4  part=xc4vsx35  speed=-10  package=ff668  synthesis_tool=XST  clock_wrapper=Clock Enables  directory=./netlist  testbench=off  simulink_period=1  sysclk_period=100  dcm_input_clock_period=100  incr_netlist=off  trim_vbits=Everywhere in SubSystem  dbl_ovrd=According to Block Masks  core_generation=According to Block Masks  run_coregen=off  deprecated_control=off  eval_field=0  block_type=sysgen
      settings: block_version=10.1.3
  Constant10 : xbsIndex_r4/Constant  arith_type=Boolean  const=1  n_bits=1  bin_pt=0  explicit_period=on  period=1  equ=P=C  opselect=C  inp2=PCIN>>17  opr=+  inp1=P  carry=CIN  dbl_ovrd=off  block_type=constant
  Constant11 : xbsIndex_r4/Constant  arith_type=Boolean  const=0  n_bits=1  bin_pt=0  explicit_period=on  period=1  equ=P=C  opselect=C  inp2=PCIN>>17  opr=+  inp1=P  carry=CIN  dbl_ovrd=off  block_type=constant
  Constant12 : xbsIndex_r4/Constant  arith_type=Boolean  const=1  n_bits=1  bin_pt=0  explicit_period=on  period=1  equ=P=C  opselect=C  inp2=PCIN>>17  opr=+  inp1=P  carry=CIN  dbl_ovrd=off  block_type=constant
  Constant2 : xbsIndex_r4/Constant  arith_type=Unsigned  const=0  n_bits=8  bin_pt=0  explicit_period=on  period=1  equ=P=C  opselect=C  inp2=PCIN>>17  opr=+  inp1=P  carry=CIN  dbl_ovrd=off  block_type=constant
  Constant3 : xbsIndex_r4/Constant  arith_type=Unsigned  const=0  n_bits=8  bin_pt=0  explicit_period=on  period=1  equ=P=C  opselect=C  inp2=PCIN>>17  opr=+  inp1=P  carry=CIN  dbl_ovrd=off  block_type=constant
  Constant9 : xbsIndex_r4/Constant  arith_type=Boolean  const=0  n_bits=1  bin_pt=0  explicit_period=on  period=1  equ=P=C  opselect=C  inp2=PCIN>>17  opr=+  inp1=P  carry=CIN  dbl_ovrd=off  block_type=constant
  Inverter : xbsIndex_r4/Inverter  en=off  latency=0  dbl_ovrd=off  block_type=inv
  Logical : xbsIndex_r4/Logical  logical_function=AND  inputs=2  en=off  latency=0  precision=Full  arith_type=Unsigned  n_bits=16  bin_pt=0  align_bp=on  dbl_ovrd=off  block_type=logical
  Logical1 : xbsIndex_r4/Logical  logical_function=OR  inputs=2  en=off  latency=1  precision=User Defined  arith_type=Unsigned  n_bits=1  bin_pt=0  align_bp=on  dbl_ovrd=off  block_type=logical
  Logical2 : xbsIndex_r4/Logical  logical_function=OR  inputs=2  en=off  latency=1  precision=User Defined  arith_type="Signed  (2's comp)"  n_bits=8  bin_pt=0  align_bp=on  dbl_ovrd=off  block_type=logical
  Median_Accumulator1 : Subsystem
  Median_Accumulator2 : Subsystem
  Median_Location1 : Subsystem
  Median_Location2 : Subsystem
  Mux : xbsIndex_r4/Mux  inputs=2  en=off  latency=0  precision=Full  arith_type=Unsigned  n_bits=16  bin_pt=14  quantization=Truncate  overflow=Wrap  dbl_ovrd=off  block_type=mux
  Mux1 : xbsIndex_r4/Mux  inputs=2  en=off  latency=0  precision=Full  arith_type="Signed  (2's comp)"  n_bits=8  bin_pt=0  quantization=Truncate  overflow=Wrap  dbl_ovrd=off  block_type=mux
  Mux2 : xbsIndex_r4/Mux  inputs=2  en=off  latency=0  precision=User Defined  arith_type="Signed  (2's comp)"  n_bits=8  bin_pt=0  quantization=Truncate  overflow=Wrap  dbl_ovrd=off  block_type=mux
  Mux3 : xbsIndex_r4/Mux  inputs=2  en=off  latency=0  precision=User Defined  arith_type="Signed  (2's comp)"  n_bits=8  bin_pt=0  quantization=Truncate  overflow=Wrap  dbl_ovrd=off  block_type=mux
  Scope{1..5} (5 alike) : Scope  NumInputPorts=2
      Scope1 settings: YMin=-5~-5  YMax=5~5
      Scope2 settings: YMin=-5~-5  YMax=5~5
      Scope3 settings: YMin=-5~-5  YMax=5~5  SaveName=ScopeData5
      Scope4 settings: YMin=-5~-5  YMax=5~5  SaveName=ScopeData7
      Scope5 settings: YMin=-5~-5  YMax=5~5  SaveName=ScopeData15
  priority_out1 : Outport  OutDataType=sfix(16)  OutScaling=2^0
  med_calc1 : Outport  Port=2  OutDataType=sfix(16)  OutScaling=2^0
  inc_out : Outport  Port=3
  wires:
  data_in/1 -> Median_Accumulator1/1(data_in), Median_Accumulator2/1(data_in)
  Median_Accumulator1/1(Out1) -> Median_Location1/1(In1), Scope5/1
  Median_Accumulator1/2(Out2) -> Median_Location1/2(In2)
  Median_Accumulator1/3(Out3) -> Median_Location1/3(In3)
  Median_Accumulator1/4(Out4) -> Median_Location1/4(In4)
  Median_Accumulator1/5(Out5) -> Median_Location1/5(In5), Scope5/2
  Median_Accumulator1/6(Out6) -> Median_Location1/6(In6)
  Median_Accumulator1/7(Out7) -> Median_Location1/7(In7)
  Median_Accumulator1/8(Out8) -> Median_Location1/8(In8)
  Median_Location1/1(Out1) -> Scope1/1, Mux2/3
  med_loc/1 -> Median_Location1/9(median_loc), Median_Location2/9(median_loc)
  Median_Accumulator2/8(Out8) -> Median_Location2/8(In8)
  Median_Accumulator2/7(Out7) -> Median_Location2/7(In7)
  Median_Accumulator2/6(Out6) -> Median_Location2/6(In6)
  Median_Accumulator2/5(Out5) -> Median_Location2/5(In5)
  Median_Accumulator2/4(Out4) -> Median_Location2/4(In4)
  Median_Accumulator2/3(Out3) -> Median_Location2/3(In3)
  Median_Accumulator2/2(Out2) -> Median_Location2/2(In2)
  Median_Accumulator2/1(Out1) -> Median_Location2/1(In1)
  Constant9/1 -> Mux/2
  Constant10/1 -> Mux/3
  Mux/1 -> Logical1/1, Mux2/1
  Inverter/1 -> Logical/1
  Logical/1 -> Mux1/1
  Constant11/1 -> Mux1/2
  Mux1/1 -> Logical1/2, Mux3/1
  Median_Location2/1(Out3) -> Mux3/3, Scope2/1, Scope4/2
  Constant12/1 -> Mux1/3
  Mux2/1 -> Logical2/1
  Mux3/1 -> Logical2/2
  Constant2/1 -> Mux2/2
  Constant3/1 -> Mux3/2
  Logical2/1 -> Scope3/2, med_calc1/1
  Logical1/1 -> priority_out1/1, Scope3/1
  Median_Accumulator2/9(inc_signal) -> inc_out/1
  Median_Location1/2(sel1) -> Mux/1, Inverter/1, Scope1/2
  Median_Location2/2(sel2) -> Logical/2, Scope2/2
  acc_reset/1 -> Median_Accumulator1/2(acc_reset), Median_Accumulator2/2(acc_reset)

== system rfi_blk_v6_casper/rfi_blk/mad_comp/Median1/Subsystem10 ==
  data_in : Inport  OutDataType=sfix(16)  OutScaling=2^0
  med_loc : Inport  Port=2  OutDataType=sfix(16)  OutScaling=2^0
  acc_reset : Inport  Port=3  OutDataType=sfix(16)  OutScaling=2^0
  " System Generator" : xbsIndex_r4/ System Generator  Tag=genX  SystemSampleTime=-1  xilinxfamily=Virtex4  part=xc4vsx35  speed=-10  package=ff668  synthesis_tool=XST  clock_wrapper=Clock Enables  directory=./netlist  testbench=off  simulink_period=1  sysclk_period=100  dcm_input_clock_period=100  incr_netlist=off  trim_vbits=Everywhere in SubSystem  dbl_ovrd=According to Block Masks  core_generation=According to Block Masks  run_coregen=off  deprecated_control=off  eval_field=0  block_type=sysgen
      settings: block_version=10.1.3
  Constant10 : xbsIndex_r4/Constant  arith_type=Boolean  const=1  n_bits=1  bin_pt=0  explicit_period=on  period=1  equ=P=C  opselect=C  inp2=PCIN>>17  opr=+  inp1=P  carry=CIN  dbl_ovrd=off  block_type=constant
  Constant11 : xbsIndex_r4/Constant  arith_type=Boolean  const=0  n_bits=1  bin_pt=0  explicit_period=on  period=1  equ=P=C  opselect=C  inp2=PCIN>>17  opr=+  inp1=P  carry=CIN  dbl_ovrd=off  block_type=constant
  Constant12 : xbsIndex_r4/Constant  arith_type=Boolean  const=1  n_bits=1  bin_pt=0  explicit_period=on  period=1  equ=P=C  opselect=C  inp2=PCIN>>17  opr=+  inp1=P  carry=CIN  dbl_ovrd=off  block_type=constant
  Constant2 : xbsIndex_r4/Constant  arith_type=Unsigned  const=0  n_bits=8  bin_pt=0  explicit_period=on  period=1  equ=P=C  opselect=C  inp2=PCIN>>17  opr=+  inp1=P  carry=CIN  dbl_ovrd=off  block_type=constant
  Constant3 : xbsIndex_r4/Constant  arith_type=Unsigned  const=0  n_bits=8  bin_pt=0  explicit_period=on  period=1  equ=P=C  opselect=C  inp2=PCIN>>17  opr=+  inp1=P  carry=CIN  dbl_ovrd=off  block_type=constant
  Constant9 : xbsIndex_r4/Constant  arith_type=Boolean  const=0  n_bits=1  bin_pt=0  explicit_period=on  period=1  equ=P=C  opselect=C  inp2=PCIN>>17  opr=+  inp1=P  carry=CIN  dbl_ovrd=off  block_type=constant
  Inverter : xbsIndex_r4/Inverter  en=off  latency=0  dbl_ovrd=off  block_type=inv
  Logical : xbsIndex_r4/Logical  logical_function=AND  inputs=2  en=off  latency=0  precision=Full  arith_type=Unsigned  n_bits=16  bin_pt=0  align_bp=on  dbl_ovrd=off  block_type=logical
  Logical1 : xbsIndex_r4/Logical  logical_function=OR  inputs=2  en=off  latency=1  precision=User Defined  arith_type=Unsigned  n_bits=1  bin_pt=0  align_bp=on  dbl_ovrd=off  block_type=logical
  Logical2 : xbsIndex_r4/Logical  logical_function=OR  inputs=2  en=off  latency=1  precision=User Defined  arith_type="Signed  (2's comp)"  n_bits=8  bin_pt=0  align_bp=on  dbl_ovrd=off  block_type=logical
  Median_Accumulator1 : Subsystem
  Median_Accumulator2 : Subsystem
  Median_Location1 : Subsystem
  Median_Location2 : Subsystem
  Mux : xbsIndex_r4/Mux  inputs=2  en=off  latency=0  precision=Full  arith_type=Unsigned  n_bits=16  bin_pt=14  quantization=Truncate  overflow=Wrap  dbl_ovrd=off  block_type=mux
  Mux1 : xbsIndex_r4/Mux  inputs=2  en=off  latency=0  precision=Full  arith_type="Signed  (2's comp)"  n_bits=8  bin_pt=0  quantization=Truncate  overflow=Wrap  dbl_ovrd=off  block_type=mux
  Mux2 : xbsIndex_r4/Mux  inputs=2  en=off  latency=0  precision=User Defined  arith_type="Signed  (2's comp)"  n_bits=8  bin_pt=0  quantization=Truncate  overflow=Wrap  dbl_ovrd=off  block_type=mux
  Mux3 : xbsIndex_r4/Mux  inputs=2  en=off  latency=0  precision=User Defined  arith_type="Signed  (2's comp)"  n_bits=8  bin_pt=0  quantization=Truncate  overflow=Wrap  dbl_ovrd=off  block_type=mux
  Scope{1..5} (5 alike) : Scope  NumInputPorts=2
      Scope1 settings: YMin=-5~-5  YMax=5~5
      Scope2 settings: YMin=-5~-5  YMax=5~5
      Scope3 settings: YMin=-5~-5  YMax=5~5  SaveName=ScopeData5
      Scope4 settings: YMin=-5~-5  YMax=5~5  SaveName=ScopeData7
      Scope5 settings: YMin=-5~-5  YMax=5~5  SaveName=ScopeData15
  priority_out1 : Outport  OutDataType=sfix(16)  OutScaling=2^0
  med_calc1 : Outport  Port=2  OutDataType=sfix(16)  OutScaling=2^0
  inc_out : Outport  Port=3
  wires:
  data_in/1 -> Median_Accumulator1/1(data_in), Median_Accumulator2/1(data_in)
  Median_Accumulator1/1(Out1) -> Median_Location1/1(In1), Scope5/1
  Median_Accumulator1/2(Out2) -> Median_Location1/2(In2)
  Median_Accumulator1/3(Out3) -> Median_Location1/3(In3)
  Median_Accumulator1/4(Out4) -> Median_Location1/4(In4)
  Median_Accumulator1/5(Out5) -> Median_Location1/5(In5), Scope5/2
  Median_Accumulator1/6(Out6) -> Median_Location1/6(In6)
  Median_Accumulator1/7(Out7) -> Median_Location1/7(In7)
  Median_Accumulator1/8(Out8) -> Median_Location1/8(In8)
  Median_Location1/1(Out1) -> Scope1/1, Mux2/3
  med_loc/1 -> Median_Location1/9(median_loc), Median_Location2/9(median_loc)
  Median_Accumulator2/8(Out8) -> Median_Location2/8(In8)
  Median_Accumulator2/7(Out7) -> Median_Location2/7(In7)
  Median_Accumulator2/6(Out6) -> Median_Location2/6(In6)
  Median_Accumulator2/5(Out5) -> Median_Location2/5(In5)
  Median_Accumulator2/4(Out4) -> Median_Location2/4(In4)
  Median_Accumulator2/3(Out3) -> Median_Location2/3(In3)
  Median_Accumulator2/2(Out2) -> Median_Location2/2(In2)
  Median_Accumulator2/1(Out1) -> Median_Location2/1(In1)
  Constant9/1 -> Mux/2
  Constant10/1 -> Mux/3
  Mux/1 -> Logical1/1, Mux2/1
  Inverter/1 -> Logical/1
  Logical/1 -> Mux1/1
  Constant11/1 -> Mux1/2
  Mux1/1 -> Logical1/2, Mux3/1
  Median_Location2/1(Out3) -> Mux3/3, Scope2/1, Scope4/2
  Constant12/1 -> Mux1/3
  Mux2/1 -> Logical2/1
  Mux3/1 -> Logical2/2
  Constant2/1 -> Mux2/2
  Constant3/1 -> Mux3/2
  Logical2/1 -> Scope3/2, med_calc1/1
  Logical1/1 -> priority_out1/1, Scope3/1
  Median_Accumulator2/9(inc_signal) -> inc_out/1
  Median_Location1/2(sel1) -> Mux/1, Inverter/1, Scope1/2
  Median_Location2/2(sel2) -> Logical/2, Scope2/2
  acc_reset/1 -> Median_Accumulator1/2(acc_reset), Median_Accumulator2/2(acc_reset)
